simulink library "lib_all"  (Simulink 7.6)
settings: Solver=ode45  SolverMode=SingleTasking  StartTime=0.0  StopTime=10.0  RelTol=1e-3
values from neighbouring files (symbols this model uses):
  map_lib_03 = [6.8679 7.0159 7.4571 8.1849 9.1892 10.456 11.97 13.71 15.655 17.781 20.063 22.474 ... (803 elements, 73x11)]    [scr_simdata.mat]    (also in scr_simdata_all.mat)
  map_lib_06 = [2.5784 2.6727 2.9588 3.4453 4.1444 5.068 6.226 7.6242 9.2634 11.139 13.24 15.552 ... (803 elements, 73x11)]    [scr_simdata.mat]    (also in scr_simdata_all.mat)
  map_lib_09 = [0.0025784 0.0026727 0.0029588 0.0034453 0.0041444 0.005068 0.006226 0.0076242 0.0092634 0.011139 0.01324 0.015552 ... (803 elements, 73x11)]    [scr_simdata.mat]    (also in scr_simdata_all.mat)
  map_lib_10 = [2.74 2.75 2.76 2.77 2.78 2.79 2.8 2.81 2.82 2.83 2.84 2.85 ... (81 elements, 1x81)]    [scr_simdata.mat]    (also in scr_simdata_all.mat)
  map_lib_11 = [1.1983 1.2027 1.207 1.2113 1.2156 1.2198 1.2241 1.2283 1.2326 1.2368 1.241 1.2451 ... (81 elements, 1x81)]    [scr_simdata.mat]    (also in scr_simdata_all.mat)
  map_lib_12 = [2.74 2.75 2.76 2.77 2.78 2.79 2.8 2.81 2.82 2.83 2.84 2.85 ... (81 elements, 1x81)]    [scr_simdata.mat]    (also in scr_simdata_all.mat)
  map_lib_13 = [1.74 1.75 1.76 1.77 1.78 1.79 1.8 1.81 1.82 1.83 1.84 1.85 ... (81 elements, 1x81)]    [scr_simdata.mat]    (also in scr_simdata_all.mat)
  map_lib_16 = [0.00048828125 1.6159 1.8962 2.0821 2.2251 2.3426 2.4433 2.5318 2.611 2.683 2.749 2.8101 ... (1419 elements, 129x11)]    [scr_simdata.mat]    (also in scr_simdata_all.mat)
  map_mr2_01 = [0 800 1600 2400 3200 4000]    [scr_simdata.mat]    (also in scr_simdata_all.mat)
  map_mr2_02 = [1.32 1.32 1.33 1.34 1.36 1.38]    [scr_simdata.mat]    (also in scr_simdata_all.mat)
  map_mr2_03 = [0 800 1600 2400 3200 4000]    [scr_simdata.mat]    (also in scr_simdata_all.mat)
  map_mr2_04 = [1.32 1.32 1.33 1.34 1.36 1.38]    [scr_simdata.mat]    (also in scr_simdata_all.mat)
  map_mr2_05 = [0 800 1600 2400 3200 4000]    [scr_simdata.mat]    (also in scr_simdata_all.mat)
  map_mr2_06 = [1.32 1.32 1.33 1.34 1.36 1.38]    [scr_simdata.mat]    (also in scr_simdata_all.mat)
  Parameter1 = 6    [scr_simdata.mat]    (also in scr_simdata_all.mat)
  Parameter101 = 30    [scr_simdata.mat]    (also in scr_simdata_all.mat)
  Parameter102 = 12    [scr_simdata.mat]    (also in scr_simdata_all.mat)
  Parameter103 = -240    [scr_simdata.mat]    (also in scr_simdata_all.mat)
  Parameter108 = 48    [scr_simdata.mat]    (also in scr_simdata_all.mat)
  Parameter120 = 16    [scr_simdata.mat]    (also in scr_simdata_all.mat)
  Parameter121 = 87    [scr_simdata.mat]    (also in scr_simdata_all.mat)
  Parameter123 = 78    [scr_simdata.mat]    (also in scr_simdata_all.mat)
  Parameter124 = 60    [scr_simdata.mat]    (also in scr_simdata_all.mat)
  Parameter125 = -480    [scr_simdata.mat]    (also in scr_simdata_all.mat)
  Parameter130 = 96    [scr_simdata.mat]    (also in scr_simdata_all.mat)
  Parameter131 = 3    [scr_simdata.mat]    (also in scr_simdata_all.mat)
  Parameter144 = 3    [scr_simdata.mat]    (also in scr_simdata_all.mat)
  Parameter18 = 128    [scr_simdata.mat]    (also in scr_simdata_all.mat)
  Parameter19 = 16    [scr_simdata.mat]    (also in scr_simdata_all.mat)
  Parameter2 = 12    [scr_simdata.mat]    (also in scr_simdata_all.mat)
  Parameter20 = 7    [scr_simdata.mat]    (also in scr_simdata_all.mat)
  Parameter21 = 4    [scr_simdata.mat]    (also in scr_simdata_all.mat)
  Parameter3 = 18    [scr_simdata.mat]    (also in scr_simdata_all.mat)
  Parameter32 = 1    [scr_simdata.mat]    (also in scr_simdata_all.mat)
  Parameter34 = 24    [scr_simdata.mat]    (also in scr_simdata_all.mat)
  Parameter4 = 138    [scr_simdata.mat]    (also in scr_simdata_all.mat)
  Parameter42 = 48    [scr_simdata.mat]    (also in scr_simdata_all.mat)
  Parameter5 = 135    [scr_simdata.mat]    (also in scr_simdata_all.mat)
  Parameter55 = 0    [scr_simdata.mat]    (also in scr_simdata_all.mat)
  Parameter56 = 0    [scr_simdata.mat]    (also in scr_simdata_all.mat)
  Parameter57 = 1    [scr_simdata.mat]    (also in scr_simdata_all.mat)
  Parameter58 = 1    [scr_simdata.mat]    (also in scr_simdata_all.mat)
  Parameter59 = 2    [scr_simdata.mat]    (also in scr_simdata_all.mat)
  Parameter6 = 132    [scr_simdata.mat]    (also in scr_simdata_all.mat)
  Parameter60 = 2    [scr_simdata.mat]    (also in scr_simdata_all.mat)
  Parameter63 = 144    [scr_simdata.mat]    (also in scr_simdata_all.mat)
  Parameter7 = 126    [scr_simdata.mat]    (also in scr_simdata_all.mat)
  Parameter76 = 64    [scr_simdata.mat]    (also in scr_simdata_all.mat)
  Parameter77 = 135    [scr_simdata.mat]    (also in scr_simdata_all.mat)
  Parameter79 = 126    [scr_simdata.mat]    (also in scr_simdata_all.mat)
  Parameter80 = 108    [scr_simdata.mat]    (also in scr_simdata_all.mat)
  Parameter81 = 0    [scr_simdata.mat]    (also in scr_simdata_all.mat)
  Parameter86 = 0    [scr_simdata.mat]    (also in scr_simdata_all.mat)
  Parameter98 = 112    [scr_simdata.mat]    (also in scr_simdata_all.mat)
  Parameter99 = 39    [scr_simdata.mat]    (also in scr_simdata_all.mat)
undefined symbols (not defined in the file or in its folder): cylvolkp_hih_map2h, cylvolkp_map2h, pk1k_map2h
libraries: simulink x2
(part 9 of 36)

== system lib_all/SubSystem660/SubSystem686/SubSystem688 ==
  note: Text99
  note: Text98
  Block1563 : Inport
  Block1564 : Inport  Port=2
  Block1565 : Constant  Value=0  OutDataTypeStr=double  SampleTime=-1
  Block1566 : Constant  Value=0  OutDataTypeStr=double  SampleTime=-1
  Block1567 : Constant  Value=720  OutDataTypeStr=double  SampleTime=-1
  Block1568 : Constant  Value=5  OutDataTypeStr=double  SampleTime=-1
  Block1569 : DataStoreRead  DataStoreName=DSM50
  Block1570 : DataStoreRead  DataStoreName=DSM35
  Block1571 : Gain  Gain=5  SaturateOnIntegerOverflow=on  OutDataTypeStr=Inherit: Same as input  ParamDataTypeStr=Inherit: Same as input
      settings: AttributesFormatString=Attribute333
  Block1572 : Gain  Gain=5  SaturateOnIntegerOverflow=on  OutDataTypeStr=Inherit: Same as input  ParamDataTypeStr=Inherit: Same as input
      settings: AttributesFormatString=Attribute334
  Block1573 : Gain  Gain=1/5  SaturateOnIntegerOverflow=on  OutDataTypeStr=Inherit: Same as input  ParamDataTypeStr=Inherit: Same as input
      settings: AttributesFormatString=Attribute335
  Block1574 : Product  Inputs=*/  OutDataTypeStr=double  SaturateOnIntegerOverflow=on  RndMeth=Zero  InputSameDT=on
  Block1575 : Rounding
  Block1576 : Rounding
  Block1577 : Sum  Inputs=+-+  OutDataTypeStr=double  RndMeth=Zero  SaturateOnIntegerOverflow=on  InputSameDT=on
  Block1578 : Sum  Inputs=-+  OutDataTypeStr=double  RndMeth=Zero  SaturateOnIntegerOverflow=on  InputSameDT=on
  SubSystem689 : Subsystem  TreatAsAtomicUnit=on  mask(***ATC.DIVISION***){bd_version=1.01.A, r0=チェック有り, r1=, r2=, r3=, r4=, r5=, r6=, r7=, r8=, r9=, c0=, c1=, c2=, c3=, c4=, c5=, c6=, c7=, c8=, c9=}
      mask prompts: bd_version "Version"
      mask help: これはAutoCからのブロックです．編集禁止．
      settings: OpenFcn=warndlg('変更禁止!');
      mask Help: web(ui_blockhelpmng(gcb));
  SubSystem690 : Subsystem  TreatAsAtomicUnit=on  mask(***ATC.DSRVEC***){bd_version=1.01.A, r0=DSM95, r1=-1, r2=, r3=, r4=, r5=, r6=, r7=, r8=, r9=, c0=, c1=, c2=, c3=, c4=, c5=, c6=, c7=, c8=, c9=}
      mask prompts: bd_version "Version"
      mask help: これはAutoCからのブロックです．編集禁止．
      settings: OpenFcn=warndlg('変更禁止!');
      mask Help: web(ui_blockhelpmng(gcb));
      mask Display: disp('ATC.DSRVEC')
  SubSystem691 : Subsystem
      settings: RTWSystemCode=Inline
  Block1587 : Outport
  Block1588 : Outport  Port=2
  wires:
  Block1576/1 -> Block1587/1
  Block1573/1 -> Block1576/1
  Block1572/1 -> Block1577/3
  Block1569/1 -> Block1577/2
  Block1570/1 -> SubSystem689/2(div)
  SubSystem690/1(Output) -> SubSystem689/1(mult)
  Block1565/1 -> SubSystem689/3(init)
  Block1568/1 -> Block1574/2
  Block1578/1 -> Block1588/1
  Block1564/1 -> SubSystem690/1(element)
  Block1567/1 -> SubSystem691/1(Block1579)
  Block1577/1 -> SubSystem691/2(Block1580)  "SigLabel39"
  Block1566/1 -> SubSystem691/3(Block1581)
  SubSystem691/1(Block1586) -> Block1574/1, Block1573/1, Block1578/2
  Block1574/1 -> Block1575/1
  Block1575/1 -> Block1571/1
  Block1571/1 -> Block1578/1
  Block1563/1 -> Block1572/1
  SubSystem689/1(Out) -> Block1577/1  "SigLabel38"

== system lib_all/SubSystem660/SubSystem686/SubSystem694 ==
  note: Text102
  note: Text101
  note: Text100
  Block1589 : Inport
  Block1590 : Inport  Port=2
  Block1591 : Inport  Port=3
  Block1592 : Constant  Value=0  OutDataTypeStr=double  SampleTime=-1
  Block1593 : Constant  OutDataTypeStr=double  SampleTime=-1
  Block1594 : Constant  OutDataTypeStr=double  SampleTime=-1
  Block1595 : Constant  Value=0  OutDataTypeStr=double  SampleTime=-1
  Block1596 : Constant  OutDataTypeStr=double  SampleTime=-1
  Block1597 : Constant  Value=143  OutDataTypeStr=double  SampleTime=-1
  Block1598 : Demux  Outputs=2
  Block1599 : Logic  Operator=NOT
  Block1600 : Mux  Inputs=2
  Block1601 : Mux  Inputs=2
  Block1602 : Sum  OutDataTypeStr=double  RndMeth=Zero  SaturateOnIntegerOverflow=on  InputSameDT=on
  Block1603 : Sum  OutDataTypeStr=double  RndMeth=Zero  SaturateOnIntegerOverflow=on  InputSameDT=on
  Block1604 : Switch  Threshold=0.5  RndMeth=Zero  SaturateOnIntegerOverflow=on  InputSameDT=on
  Block1605 : Switch  Threshold=0.5  RndMeth=Zero  SaturateOnIntegerOverflow=on  InputSameDT=on
  Block1606 : Switch  Threshold=0.5  RndMeth=Zero  SaturateOnIntegerOverflow=on  InputSameDT=on
  SubSystem695 : Subsystem
  SubSystem696 : Subsystem
  SubSystem697 : Subsystem
  SubSystem698 : Subsystem  TreatAsAtomicUnit=on
  SubSystem707 : Subsystem  TreatAsAtomicUnit=on
  SubSystem717 : Subsystem  TreatAsAtomicUnit=on
  Block1651 : Outport
  Block1652 : Outport  Port=2
  wires:
  Block1589/1 -> SubSystem695/1(In1), Block1602/2, Block1600/1
  Block1597/1 -> SubSystem695/2(In2), Block1601/1
  Block1590/1 -> SubSystem696/1(In1), SubSystem697/1(In1)
  Block1592/1 -> SubSystem696/2(In2)
  SubSystem696/1(Out1) -> Block1604/2, SubSystem698/Enable
  SubSystem698/1(Block1620) -> Block1604/1
  Block1593/1 -> SubSystem697/2(In2)
  SubSystem697/1(Out1) -> Block1605/2, SubSystem707/Enable, Block1599/1
  SubSystem707/1(Block1635) -> Block1605/1
  Block1599/1 -> SubSystem717/Enable
  Block1605/1 -> Block1604/3
  SubSystem717/1(Block1650) -> Block1605/3
  Block1598/1 -> Block1651/1
  Block1598/2 -> Block1652/1
  Block1604/1 -> Block1598/1
  SubSystem695/1(Out1) -> Block1606/2
  Block1594/1 -> Block1602/1
  Block1595/1 -> Block1601/2
  Block1601/1 -> Block1606/3
  Block1602/1 -> Block1600/2
  Block1600/1 -> Block1606/1
  Block1591/1 -> SubSystem698/2(Block1608), SubSystem707/2(Block1622), SubSystem717/2(Block1637)
  Block1606/1 -> Block1603/2
  Block1596/1 -> Block1603/1
  Block1603/1 -> SubSystem698/1(Block1607), SubSystem707/1(Block1621), SubSystem717/1(Block1636)

== system lib_all/SubSystem7/SubSystem134/SubSystem135 ==
  note: ***編集禁止***
  In1 : Inport
  In2 : Inport  Port=2
  RO1 : RelationalOperator  Operator===  InputSameDT=on
  single1 : DataTypeConversion  OutDataTypeStr=single  SaturateOnIntegerOverflow=on  RndMeth=Zero
  single2 : DataTypeConversion  OutDataTypeStr=single  SaturateOnIntegerOverflow=on  RndMeth=Zero
  Out1 : Outport
  wires:
  RO1/1 -> Out1/1
  In2/1 -> single2/1
  In1/1 -> single1/1
  single1/1 -> RO1/1
  single2/1 -> RO1/2

== system lib_all/SubSystem7/SubSystem134/SubSystem136 ==
  note: Text21
  Block317 : Inport
  Block318 : EnablePort
  Block319 : Constant  Value=Parameter18  OutDataTypeStr=double  SampleTime=-1
  SubSystem137 : Subsystem  TreatAsAtomicUnit=on
      settings: RTWSystemCode=Inline
  SubSystem139 : Subsystem
  SubSystem149 : Subsystem  TreatAsAtomicUnit=on
  wires:
  Block317/1 -> SubSystem149/2(Block344), SubSystem139/3(Block325)
  Block319/1 -> SubSystem137/1(N)
  SubSystem137/1(action) -> SubSystem149/Trigger
  SubSystem137/2(i) -> SubSystem149/1(Block343)
  SubSystem149/1(Block361) -> SubSystem139/1(Block323)
  SubSystem149/2(Block362) -> SubSystem139/2(Block324)

== system lib_all/SubSystem7/SubSystem134/SubSystem159 ==
  note: ***編集禁止***
  element : Inport
  Inside_DSR : DataStoreRead  DataStoreName=DSM374
  Selector : Selector  IndexOptions=Index vector (port)  Indices=[1 3]  OutputSizes=1
  Output : Outport
  wires:
  Selector/1 -> Output/1
  element/1 -> Selector/2
  Inside_DSR/1 -> Selector/1

== system lib_all/SubSystem7/SubSystem134/SubSystem160 ==
  note: ***編集禁止***
  input : Inport
  element : Inport  Port=2
  Assignment : Assignment  IndexOptions=Index vector (port)  Indices=1  OutputSizes=[1 1]
  Inside_DSR : DataStoreRead  DataStoreName=DSM374
  Inside_DSW : DataStoreWrite  DataStoreName=DSM374
  wires:
  element/1 -> Assignment/3
  input/1 -> Assignment/2
  Inside_DSR/1 -> Assignment/1
  Assignment/1 -> Inside_DSW/1

== system lib_all/SubSystem7/SubSystem161/SubSystem162 ==
  note: ***編集禁止***
  In1 : Inport
  In2 : Inport  Port=2
  LO1 : Logic  Operator=OR
  RO1 : RelationalOperator  InputSameDT=on
  RO2 : RelationalOperator  Operator===  InputSameDT=on
  single1 : DataTypeConversion  OutDataTypeStr=single  SaturateOnIntegerOverflow=on  RndMeth=Zero
  single2 : DataTypeConversion  OutDataTypeStr=single  SaturateOnIntegerOverflow=on  RndMeth=Zero
  Out1 : Outport
  wires:
  In2/1 -> single2/1
  In1/1 -> single1/1
  single1/1 -> RO1/1, RO2/1
  single2/1 -> RO1/2, RO2/2
  RO1/1 -> LO1/1
  RO2/1 -> LO1/2
  LO1/1 -> Out1/1

== system lib_all/SubSystem7/SubSystem161/SubSystem163 ==
  note: ***編集禁止***
  In1 : Inport
  In2 : Inport  Port=2
  RO1 : RelationalOperator  Operator===  InputSameDT=on
  single1 : DataTypeConversion  OutDataTypeStr=single  SaturateOnIntegerOverflow=on  RndMeth=Zero
  single2 : DataTypeConversion  OutDataTypeStr=single  SaturateOnIntegerOverflow=on  RndMeth=Zero
  Out1 : Outport
  wires:
  RO1/1 -> Out1/1
  In2/1 -> single2/1
  In1/1 -> single1/1
  single1/1 -> RO1/1
  single2/1 -> RO1/2

== system lib_all/SubSystem7/SubSystem161/SubSystem164 ==
  note: Text25
  Block367 : EnablePort
  Block368 : Constant  Value=Parameter131  OutDataTypeStr=double  SampleTime=-1
  SubSystem165 : Subsystem  TreatAsAtomicUnit=on
      settings: RTWSystemCode=Inline
  SubSystem167 : Subsystem  TreatAsAtomicUnit=on
      settings: RTWSystemCode=Inline
  SubSystem169 : Subsystem  TreatAsAtomicUnit=on
  SubSystem173 : Subsystem  TreatAsAtomicUnit=on
  SubSystem175 : Subsystem  Priority=2
      settings: AttributesFormatString=Attribute80
  wires:
  Block368/1 -> SubSystem165/1(N), SubSystem167/1(N)
  SubSystem165/1(action) -> SubSystem169/Trigger
  SubSystem165/2(i) -> SubSystem169/1(Block375)
  SubSystem167/1(action) -> SubSystem173/Trigger
  SubSystem167/2(i) -> SubSystem173/1(Block379)

== system lib_all/SubSystem7/SubSystem9/SubSystem10 ==
  note: ***編集禁止***
  In1 : Inport
  In2 : Inport  Port=2
  LO1 : Logic
  RO1 : RelationalOperator  Operator=<  InputSameDT=on
  RO2 : RelationalOperator  Operator=~=  InputSameDT=on
  single1 : DataTypeConversion  OutDataTypeStr=single  SaturateOnIntegerOverflow=on  RndMeth=Zero
  single2 : DataTypeConversion  OutDataTypeStr=single  SaturateOnIntegerOverflow=on  RndMeth=Zero
  Out1 : Outport
  wires:
  In2/1 -> single2/1
  In1/1 -> single1/1
  single1/1 -> RO1/1, RO2/1
  single2/1 -> RO1/2, RO2/2
  RO1/1 -> LO1/1
  RO2/1 -> LO1/2
  LO1/1 -> Out1/1

== system lib_all/SubSystem7/SubSystem9/SubSystem11 ==
  Block16 : Inport
  Block17 : Constant  Value=Parameter120  OutDataTypeStr=double  SampleTime=-1
  Block18 : Constant  Value=Parameter80  OutDataTypeStr=double  SampleTime=-1
  Block19 : Constant  Value=Parameter102  OutDataTypeStr=double  SampleTime=-1
  Block20 : Constant  Value=Parameter124  OutDataTypeStr=double  SampleTime=-1
  Block21 : Constant  Value=Parameter76  OutDataTypeStr=double  SampleTime=-1
  Block22 : Constant  Value=Parameter98  OutDataTypeStr=double  SampleTime=-1
  Block23 : MultiPortSwitch  zeroidx=off  RndMeth=Zero  SaturateOnIntegerOverflow=on  InputSameDT=on
  Block24 : Mux  Inputs=2
  Block25 : Mux  Inputs=2
  Block26 : Mux  Inputs=2
  Block27 : Outport
  wires:
  Block23/1 -> Block27/1
  Block16/1 -> Block23/1
  Block18/1 -> Block24/1
  Block19/1 -> Block25/1
  Block20/1 -> Block26/1
  Block17/1 -> Block26/2
  Block22/1 -> Block25/2
  Block24/1 -> Block23/2
  Block26/1 -> Block23/4
  Block25/1 -> Block23/3
  Block21/1 -> Block24/2

== system lib_all/SubSystem7/SubSystem9/SubSystem12 ==
  note: ***編集禁止***
  element : Inport
  Inside_DSR : DataStoreRead  DataStoreName=DSM374
  Selector : Selector  IndexOptions=Index vector (port)  Indices=[1 3]  OutputSizes=1
  Output : Outport
  wires:
  Selector/1 -> Output/1
  element/1 -> Selector/2
  Inside_DSR/1 -> Selector/1

== system lib_all/SubSystem7/SubSystem9/SubSystem13 ==
  Block28 : Inport
  Block29 : Inport  Port=2
  Block30 : Inport  Port=3
  Block31 : Inport  Port=4
  Block32 : EnablePort
  Block33 : Constant  Value=0  OutDataTypeStr=double  SampleTime=-1
  Block34 : Constant  OutDataTypeStr=double  SampleTime=-1
  Block35 : Constant  OutDataTypeStr=double  SampleTime=-1
  Block36 : Constant  Value=0  OutDataTypeStr=double  SampleTime=-1
  Block37 : Sum  OutDataTypeStr=double  RndMeth=Zero  SaturateOnIntegerOverflow=on  InputSameDT=on
  Block38 : Sum  OutDataTypeStr=double  RndMeth=Zero  SaturateOnIntegerOverflow=on  InputSameDT=on
  SubSystem120 : Subsystem
  SubSystem127 : Subsystem
  SubSystem14 : Subsystem  TreatAsAtomicUnit=on
      settings: AttributesFormatString=Attribute16  RTWFcnNameOpts=Use subsystem name
  SubSystem67 : Subsystem  TreatAsAtomicUnit=on
      settings: AttributesFormatString=Attribute35  RTWFcnNameOpts=Use subsystem name
  wires:
  Block38/1 -> SubSystem127/3(Block299), SubSystem120/3(Block285)
  Block35/1 -> Block38/2
  Block31/1 -> Block38/1
  Block28/1 -> SubSystem67/1(Block161), SubSystem14/1(Block39), Block37/1
  Block30/1 -> SubSystem14/2(Block40)
  Block29/1 -> SubSystem67/2(Block162)
  Block36/1 -> SubSystem14/4(Block42), SubSystem14/3(Block41)
  Block33/1 -> SubSystem67/4(Block164), SubSystem67/3(Block163)
  SubSystem14/1(Block160) -> SubSystem120/1(Block283)
  Block37/1 -> SubSystem120/2(Block284), SubSystem127/2(Block298)
  SubSystem67/1(Block282) -> SubSystem127/1(Block297)
  Block34/1 -> Block37/2

== system lib_all/SubSystem727/SubSystem740/SubSystem742 ==
  note: ***編集禁止***
  In1 : Inport
  In2 : Inport  Port=2
  RO1 : RelationalOperator  Operator===  InputSameDT=on
  single1 : DataTypeConversion  OutDataTypeStr=single  SaturateOnIntegerOverflow=on  RndMeth=Zero
  single2 : DataTypeConversion  OutDataTypeStr=single  SaturateOnIntegerOverflow=on  RndMeth=Zero
  Out1 : Outport
  wires:
  RO1/1 -> Out1/1
  In2/1 -> single2/1
  In1/1 -> single1/1
  single1/1 -> RO1/1
  single2/1 -> RO1/2

== system lib_all/SubSystem727/SubSystem740/SubSystem743 ==
  note: Text110
  Block1732 : Inport
  Block1733 : Inport  Port=2
  Block1734 : Constant  Value=0  OutDataTypeStr=double  SampleTime=-1
  Block1735 : Constant  Value=720  OutDataTypeStr=double  SampleTime=-1
  Block1736 : Gain  Gain=5  SaturateOnIntegerOverflow=on  OutDataTypeStr=Inherit: Same as input  ParamDataTypeStr=Inherit: Same as input
      settings: AttributesFormatString=Attribute353
  SubSystem744 : Subsystem
      settings: RTWFcnNameOpts=Use subsystem name
  SubSystem749 : Subsystem
      settings: RTWSystemCode=Inline
  Block1762 : Outport
  wires:
  Block1736/1 -> SubSystem749/2(Block1757)
  SubSystem744/1(Block1755) -> Block1736/1
  Block1733/1 -> SubSystem744/2(Block1738)
  Block1732/1 -> SubSystem744/1(Block1737)
  SubSystem749/1(Block1761) -> Block1762/1
  Block1735/1 -> SubSystem749/1(Block1756)
  Block1734/1 -> SubSystem749/3(Block1758)

== system lib_all/SubSystem727/SubSystem740/SubSystem751 ==
  Block1763 : Inport
  Block1764 : Inport  Port=2
  Block1765 : Inport  Port=3
  Block1766 : EnablePort
  Block1767 : DataStoreWrite  DataStoreName=DSM12
  Block1768 : DataStoreWrite  DataStoreName=DSM14
  Block1769 : DataStoreWrite  DataStoreName=DSM13
  wires:
  Block1763/1 -> Block1767/1
  Block1765/1 -> Block1769/1
  Block1764/1 -> Block1768/1

== system lib_all/SubSystem727/SubSystem740/SubSystem752 ==
  note: Text111
  Block1770 : Inport
  Block1771 : Inport  Port=2
  Block1772 : Constant  Value=360  OutDataTypeStr=double  SampleTime=-1
  Block1773 : Constant  Value=360  OutDataTypeStr=double  SampleTime=-1
  Block1774 : Sum  Inputs=+-  OutDataTypeStr=double  RndMeth=Zero  SaturateOnIntegerOverflow=on  InputSameDT=on
  Block1775 : Switch  Threshold=0.5  RndMeth=Zero  SaturateOnIntegerOverflow=on  InputSameDT=on
  Block1776 : Lookup2D  RowIndex=map_lib_05  ColumnIndex=map_lib_04  Table=map_lib_06  SaturateOnIntegerOverflow=on  InputSameDT=on
      settings: AttributesFormatString=<%<OutputValues>>\nAttribute362
  SubSystem753 : Subsystem
  Block1777 : Outport
  wires:
  Block1771/1 -> SubSystem753/1(In1), Block1775/3, Block1774/1
  Block1773/1 -> SubSystem753/2(In2)
  SubSystem753/1(Out1) -> Block1775/2
  Block1774/1 -> Block1775/1
  Block1772/1 -> Block1774/2
  Block1776/1 -> Block1777/1
  Block1770/1 -> Block1776/2
  Block1775/1 -> Block1776/1  "SigLabel45"

== system lib_all/SubSystem727/SubSystem754/SubSystem756 ==
  note: Text115
  note: Text114
  Block1788 : Inport
  Block1789 : Inport  Port=2
  Block1790 : Constant  Value=0  OutDataTypeStr=double  SampleTime=-1
  Block1791 : Constant  Value=0  OutDataTypeStr=double  SampleTime=-1
  Block1792 : Constant  Value=720  OutDataTypeStr=double  SampleTime=-1
  Block1793 : Constant  Value=5  OutDataTypeStr=double  SampleTime=-1
  Block1794 : DataStoreRead  DataStoreName=DSM50
  Block1795 : DataStoreRead  DataStoreName=DSM35
  Block1796 : Gain  Gain=5  SaturateOnIntegerOverflow=on  OutDataTypeStr=Inherit: Same as input  ParamDataTypeStr=Inherit: Same as input
      settings: AttributesFormatString=Attribute370
  Block1797 : Gain  Gain=5  SaturateOnIntegerOverflow=on  OutDataTypeStr=Inherit: Same as input  ParamDataTypeStr=Inherit: Same as input
      settings: AttributesFormatString=Attribute371
  Block1798 : Gain  Gain=1/5  SaturateOnIntegerOverflow=on  OutDataTypeStr=Inherit: Same as input  ParamDataTypeStr=Inherit: Same as input
      settings: AttributesFormatString=Attribute372
  Block1799 : Product  Inputs=*/  OutDataTypeStr=double  SaturateOnIntegerOverflow=on  RndMeth=Zero  InputSameDT=on
  Block1800 : Rounding
  Block1801 : Rounding
  Block1802 : Sum  Inputs=+-+  OutDataTypeStr=double  RndMeth=Zero  SaturateOnIntegerOverflow=on  InputSameDT=on
  Block1803 : Sum  Inputs=-+  OutDataTypeStr=double  RndMeth=Zero  SaturateOnIntegerOverflow=on  InputSameDT=on
  SubSystem757 : Subsystem  TreatAsAtomicUnit=on  mask(***ATC.DIVISION***){bd_version=1.01.A, r0=チェック有り, r1=, r2=, r3=, r4=, r5=, r6=, r7=, r8=, r9=, c0=, c1=, c2=, c3=, c4=, c5=, c6=, c7=, c8=, c9=}
      mask prompts: bd_version "Version"
      mask help: これはAutoCからのブロックです．編集禁止．
      settings: OpenFcn=warndlg('変更禁止!');
      mask Help: web(ui_blockhelpmng(gcb));
  SubSystem758 : Subsystem  TreatAsAtomicUnit=on  mask(***ATC.DSRVEC***){bd_version=1.01.A, r0=DSM95, r1=-1, r2=, r3=, r4=, r5=, r6=, r7=, r8=, r9=, c0=, c1=, c2=, c3=, c4=, c5=, c6=, c7=, c8=, c9=}
      mask prompts: bd_version "Version"
      mask help: これはAutoCからのブロックです．編集禁止．
      settings: OpenFcn=warndlg('変更禁止!');
      mask Help: web(ui_blockhelpmng(gcb));
      mask Display: disp('ATC.DSRVEC')
  SubSystem759 : Subsystem
      settings: RTWSystemCode=Inline
  Block1812 : Outport
  Block1813 : Outport  Port=2
  wires:
  Block1801/1 -> Block1812/1
  Block1798/1 -> Block1801/1
  Block1797/1 -> Block1802/3
  Block1794/1 -> Block1802/2
  Block1795/1 -> SubSystem757/2(div)
  SubSystem758/1(Output) -> SubSystem757/1(mult)
  Block1790/1 -> SubSystem757/3(init)
  Block1793/1 -> Block1799/2
  Block1803/1 -> Block1813/1
  Block1789/1 -> SubSystem758/1(element)
  Block1792/1 -> SubSystem759/1(Block1804)
  Block1802/1 -> SubSystem759/2(Block1805)  "SigLabel47"
  Block1791/1 -> SubSystem759/3(Block1806)
  SubSystem759/1(Block1811) -> Block1799/1, Block1798/1, Block1803/2
  Block1799/1 -> Block1800/1
  Block1800/1 -> Block1796/1
  Block1796/1 -> Block1803/1
  Block1788/1 -> Block1797/1
  SubSystem757/1(Out) -> Block1802/1  "SigLabel46"

== system lib_all/SubSystem727/SubSystem754/SubSystem762 ==
  note: Text118
  note: Text117
  note: Text116
  Block1814 : Inport
  Block1815 : Inport  Port=2
  Block1816 : Inport  Port=3
  Block1817 : Constant  Value=0  OutDataTypeStr=double  SampleTime=-1
  Block1818 : Constant  OutDataTypeStr=double  SampleTime=-1
  Block1819 : Constant  OutDataTypeStr=double  SampleTime=-1
  Block1820 : Constant  Value=0  OutDataTypeStr=double  SampleTime=-1
  Block1821 : Constant  OutDataTypeStr=double  SampleTime=-1
  Block1822 : Constant  Value=143  OutDataTypeStr=double  SampleTime=-1
  Block1823 : Demux  Outputs=2
  Block1824 : Logic  Operator=NOT
  Block1825 : Mux  Inputs=2
  Block1826 : Mux  Inputs=2
  Block1827 : Sum  OutDataTypeStr=double  RndMeth=Zero  SaturateOnIntegerOverflow=on  InputSameDT=on
  Block1828 : Sum  OutDataTypeStr=double  RndMeth=Zero  SaturateOnIntegerOverflow=on  InputSameDT=on
  Block1829 : Switch  Threshold=0.5  RndMeth=Zero  SaturateOnIntegerOverflow=on  InputSameDT=on
  Block1830 : Switch  Threshold=0.5  RndMeth=Zero  SaturateOnIntegerOverflow=on  InputSameDT=on
  Block1831 : Switch  Threshold=0.5  RndMeth=Zero  SaturateOnIntegerOverflow=on  InputSameDT=on
  SubSystem763 : Subsystem
  SubSystem764 : Subsystem
  SubSystem765 : Subsystem
  SubSystem766 : Subsystem  TreatAsAtomicUnit=on
  SubSystem775 : Subsystem  TreatAsAtomicUnit=on
  SubSystem785 : Subsystem  TreatAsAtomicUnit=on
  Block1876 : Outport
  Block1877 : Outport  Port=2
  wires:
  Block1814/1 -> SubSystem763/1(In1), Block1827/2, Block1825/1
  Block1822/1 -> SubSystem763/2(In2), Block1826/1
  Block1815/1 -> SubSystem764/1(In1), SubSystem765/1(In1)
  Block1817/1 -> SubSystem764/2(In2)
  SubSystem764/1(Out1) -> Block1829/2, SubSystem766/Enable
  SubSystem766/1(Block1845) -> Block1829/1
  Block1818/1 -> SubSystem765/2(In2)
  SubSystem765/1(Out1) -> Block1830/2, SubSystem775/Enable, Block1824/1
  SubSystem775/1(Block1860) -> Block1830/1
  Block1824/1 -> SubSystem785/Enable
  Block1830/1 -> Block1829/3
  SubSystem785/1(Block1875) -> Block1830/3
  Block1823/1 -> Block1876/1
  Block1823/2 -> Block1877/1
  Block1829/1 -> Block1823/1
  SubSystem763/1(Out1) -> Block1831/2
  Block1819/1 -> Block1827/1
  Block1820/1 -> Block1826/2
  Block1826/1 -> Block1831/3
  Block1827/1 -> Block1825/2
  Block1825/1 -> Block1831/1
  Block1816/1 -> SubSystem766/2(Block1833), SubSystem775/2(Block1847), SubSystem785/2(Block1862)
  Block1831/1 -> Block1828/2
  Block1821/1 -> Block1828/1
  Block1828/1 -> SubSystem766/1(Block1832), SubSystem775/1(Block1846), SubSystem785/1(Block1861)

== system lib_all/SubSystem800/SubSystem803/SubSystem804 ==
  note: Text130
  Block1929 : Inport
  Block1930 : Inport  Port=2
  Block1931 : Inport  Port=3
  Block{1932,1934,1936..1937} (4 alike) : Constant  OutDataTypeStr=double  SampleTime=-1
  Block1933 : Constant  Value=0  OutDataTypeStr=double  SampleTime=-1
  Block1935 : Constant  Value=0  OutDataTypeStr=double  SampleTime=-1
  Block1943 : Sum  OutDataTypeStr=double  RndMeth=Zero  SaturateOnIntegerOverflow=on  InputSameDT=on
  Block1944 : Sum  Inputs=+++  OutDataTypeStr=double  RndMeth=Zero  SaturateOnIntegerOverflow=on  InputSameDT=on
  Block1945 : Sum  Inputs=+++  OutDataTypeStr=double  RndMeth=Zero  SaturateOnIntegerOverflow=on  InputSameDT=on
  Block1946 : Sum  Inputs=--+  OutDataTypeStr=double  RndMeth=Zero  SaturateOnIntegerOverflow=on  InputSameDT=on
  Block1947 : Sum  Inputs=--+  OutDataTypeStr=double  RndMeth=Zero  SaturateOnIntegerOverflow=on  InputSameDT=on
  Block{1948..1951} (4 alike) : Switch  Threshold=0.5  RndMeth=Zero  SaturateOnIntegerOverflow=on  InputSameDT=on
  SubSystem805 : Subsystem
  SubSystem806 : Subsystem
  SubSystem807 : Subsystem
  SubSystem808 : Subsystem
  Block1952 : Outport
  wires:
  Block1943/1 -> Block1946/3, SubSystem807/2(In2), Block1950/3, SubSystem805/2(In2), Block1944/3
  Block1949/1 -> Block1952/1
  Block1931/1 -> Block1943/2
  Block1930/1 -> Block1943/1
  Block1951/1 -> Block1949/3
  Block1937/1 -> Block1947/2
  Block1947/1 -> Block1951/1
  Block1950/1 -> SubSystem808/2(In2), Block1951/3, Block1947/3
  [TagName14] -> SubSystem808/1(In1)
  SubSystem808/1(Out1) -> Block1951/2
  [TagName14] -> Block1947/1, Block1946/1
  Block1936/1 -> Block1946/2
  Block1946/1 -> Block1950/1
  [TagName14] -> SubSystem807/1(In1)
  SubSystem807/1(Out1) -> Block1950/2
  Block1948/1 -> Block1949/1
  Block1934/1 -> Block1945/2
  Block1945/1 -> Block1948/1
  Block1935/1 -> SubSystem806/1(In1)
  SubSystem806/1(Out1) -> Block1948/2
  [TagName14] -> Block1945/1, Block1944/1
  Block1932/1 -> Block1944/2
  Block1944/1 -> SubSystem806/2(In2), Block1948/3, Block1945/3
  Block1933/1 -> SubSystem805/1(In1)
  SubSystem805/1(Out1) -> Block1949/2
  Block1929/1 -> [TagName14]

== system lib_all/SubSystem800/SubSystem803/SubSystem809 ==
  note: Text131
  Block1953 : Inport
  Block1954 : Inport  Port=2
  Block1955 : Inport  Port=3
  Block{1956,1958,1960..1961} (4 alike) : Constant  OutDataTypeStr=double  SampleTime=-1
  Block1957 : Constant  Value=0  OutDataTypeStr=double  SampleTime=-1
  Block1959 : Constant  Value=0  OutDataTypeStr=double  SampleTime=-1
  Block1967 : Sum  OutDataTypeStr=double  RndMeth=Zero  SaturateOnIntegerOverflow=on  InputSameDT=on
  Block1968 : Sum  Inputs=+++  OutDataTypeStr=double  RndMeth=Zero  SaturateOnIntegerOverflow=on  InputSameDT=on
  Block1969 : Sum  Inputs=+++  OutDataTypeStr=double  RndMeth=Zero  SaturateOnIntegerOverflow=on  InputSameDT=on
  Block1970 : Sum  Inputs=--+  OutDataTypeStr=double  RndMeth=Zero  SaturateOnIntegerOverflow=on  InputSameDT=on
  Block1971 : Sum  Inputs=--+  OutDataTypeStr=double  RndMeth=Zero  SaturateOnIntegerOverflow=on  InputSameDT=on
  Block{1972..1975} (4 alike) : Switch  Threshold=0.5  RndMeth=Zero  SaturateOnIntegerOverflow=on  InputSameDT=on
  SubSystem810 : Subsystem
  SubSystem811 : Subsystem
  SubSystem812 : Subsystem
  SubSystem813 : Subsystem
  Block1976 : Outport
  wires:
  Block1967/1 -> Block1970/3, SubSystem812/2(In2), Block1974/3, SubSystem810/2(In2), Block1968/3
  Block1973/1 -> Block1976/1
  Block1955/1 -> Block1967/2
  Block1954/1 -> Block1967/1
  Block1975/1 -> Block1973/3
  Block1961/1 -> Block1971/2
  Block1971/1 -> Block1975/1
  Block1974/1 -> SubSystem813/2(In2), Block1975/3, Block1971/3
  [TagName14] -> SubSystem813/1(In1)
  SubSystem813/1(Out1) -> Block1975/2
  [TagName14] -> Block1971/1, Block1970/1
  Block1960/1 -> Block1970/2
  Block1970/1 -> Block1974/1
  [TagName14] -> SubSystem812/1(In1)
  SubSystem812/1(Out1) -> Block1974/2
  Block1972/1 -> Block1973/1
  Block1958/1 -> Block1969/2
  Block1969/1 -> Block1972/1
  Block1959/1 -> SubSystem811/1(In1)
  SubSystem811/1(Out1) -> Block1972/2
  [TagName14] -> Block1969/1, Block1968/1
  Block1956/1 -> Block1968/2
  Block1968/1 -> SubSystem811/2(In2), Block1972/3, Block1969/3
  Block1957/1 -> SubSystem810/1(In1)
  SubSystem810/1(Out1) -> Block1973/2
  Block1953/1 -> [TagName14]

== system lib_all/SubSystem800/SubSystem803/SubSystem814 ==
  note: Text132
  Block1977 : Inport
  Block1978 : Inport  Port=2
  Block1979 : Inport  Port=3
  Block{1980,1982,1984..1985} (4 alike) : Constant  OutDataTypeStr=double  SampleTime=-1
  Block1981 : Constant  Value=0  OutDataTypeStr=double  SampleTime=-1
  Block1983 : Constant  Value=0  OutDataTypeStr=double  SampleTime=-1
  Block1991 : Sum  OutDataTypeStr=double  RndMeth=Zero  SaturateOnIntegerOverflow=on  InputSameDT=on
  Block1992 : Sum  Inputs=+++  OutDataTypeStr=double  RndMeth=Zero  SaturateOnIntegerOverflow=on  InputSameDT=on
  Block1993 : Sum  Inputs=+++  OutDataTypeStr=double  RndMeth=Zero  SaturateOnIntegerOverflow=on  InputSameDT=on
  Block1994 : Sum  Inputs=--+  OutDataTypeStr=double  RndMeth=Zero  SaturateOnIntegerOverflow=on  InputSameDT=on
  Block1995 : Sum  Inputs=--+  OutDataTypeStr=double  RndMeth=Zero  SaturateOnIntegerOverflow=on  InputSameDT=on
  Block{1996..1999} (4 alike) : Switch  Threshold=0.5  RndMeth=Zero  SaturateOnIntegerOverflow=on  InputSameDT=on
  SubSystem815 : Subsystem
  SubSystem816 : Subsystem
  SubSystem817 : Subsystem
  SubSystem818 : Subsystem
  Block2000 : Outport
  wires:
  Block1991/1 -> Block1994/3, SubSystem817/2(In2), Block1998/3, SubSystem815/2(In2), Block1992/3
  Block1997/1 -> Block2000/1
  Block1979/1 -> Block1991/2
  Block1978/1 -> Block1991/1
  Block1999/1 -> Block1997/3
  Block1985/1 -> Block1995/2
  Block1995/1 -> Block1999/1
  Block1998/1 -> SubSystem818/2(In2), Block1999/3, Block1995/3
  [TagName14] -> SubSystem818/1(In1)
  SubSystem818/1(Out1) -> Block1999/2
  [TagName14] -> Block1995/1, Block1994/1
  Block1984/1 -> Block1994/2
  Block1994/1 -> Block1998/1
  [TagName14] -> SubSystem817/1(In1)
  SubSystem817/1(Out1) -> Block1998/2
  Block1996/1 -> Block1997/1
  Block1982/1 -> Block1993/2
  Block1993/1 -> Block1996/1
  Block1983/1 -> SubSystem816/1(In1)
  SubSystem816/1(Out1) -> Block1996/2
  [TagName14] -> Block1993/1, Block1992/1
  Block1980/1 -> Block1992/2
  Block1992/1 -> SubSystem816/2(In2), Block1996/3, Block1993/3
  Block1981/1 -> SubSystem815/1(In1)
  SubSystem815/1(Out1) -> Block1997/2
  Block1977/1 -> [TagName14]

== system lib_all/SubSystem800/SubSystem819/SubSystem821 ==
  note: ***編集禁止***
  In1 : Inport
  In2 : Inport  Port=2
  RO1 : RelationalOperator  Operator===  InputSameDT=on
  single1 : DataTypeConversion  OutDataTypeStr=single  SaturateOnIntegerOverflow=on  RndMeth=Zero
  single2 : DataTypeConversion  OutDataTypeStr=single  SaturateOnIntegerOverflow=on  RndMeth=Zero
  Out1 : Outport
  wires:
  RO1/1 -> Out1/1
  In2/1 -> single2/1
  In1/1 -> single1/1
  single1/1 -> RO1/1
  single2/1 -> RO1/2

== system lib_all/SubSystem800/SubSystem819/SubSystem822 ==
  note: ***編集禁止***
  In1 : Inport
  In2 : Inport  Port=2
  RO1 : RelationalOperator  Operator===  InputSameDT=on
  single1 : DataTypeConversion  OutDataTypeStr=single  SaturateOnIntegerOverflow=on  RndMeth=Zero
  single2 : DataTypeConversion  OutDataTypeStr=single  SaturateOnIntegerOverflow=on  RndMeth=Zero
  Out1 : Outport
  wires:
  RO1/1 -> Out1/1
  In2/1 -> single2/1
  In1/1 -> single1/1
  single1/1 -> RO1/1
  single2/1 -> RO1/2

== system lib_all/SubSystem800/SubSystem819/SubSystem823 ==
  note: ***編集禁止***
  In1 : Inport
  In2 : Inport  Port=2
  RO1 : RelationalOperator  Operator===  InputSameDT=on
  single1 : DataTypeConversion  OutDataTypeStr=single  SaturateOnIntegerOverflow=on  RndMeth=Zero
  single2 : DataTypeConversion  OutDataTypeStr=single  SaturateOnIntegerOverflow=on  RndMeth=Zero
  Out1 : Outport
  wires:
  RO1/1 -> Out1/1
  In2/1 -> single2/1
  In1/1 -> single1/1
  single1/1 -> RO1/1
  single2/1 -> RO1/2

== system lib_all/SubSystem800/SubSystem819/SubSystem824 ==
  note: ***編集禁止***
  In1 : Inport
  In2 : Inport  Port=2
  RO1 : RelationalOperator  Operator===  InputSameDT=on
  single1 : DataTypeConversion  OutDataTypeStr=single  SaturateOnIntegerOverflow=on  RndMeth=Zero
  single2 : DataTypeConversion  OutDataTypeStr=single  SaturateOnIntegerOverflow=on  RndMeth=Zero
  Out1 : Outport
  wires:
  RO1/1 -> Out1/1
  In2/1 -> single2/1
  In1/1 -> single1/1
  single1/1 -> RO1/1
  single2/1 -> RO1/2

== system lib_all/SubSystem800/SubSystem819/SubSystem825 ==
  note: ***編集禁止***
  In1 : Inport
  In2 : Inport  Port=2
  RO1 : RelationalOperator  Operator===  InputSameDT=on
  single1 : DataTypeConversion  OutDataTypeStr=single  SaturateOnIntegerOverflow=on  RndMeth=Zero
  single2 : DataTypeConversion  OutDataTypeStr=single  SaturateOnIntegerOverflow=on  RndMeth=Zero
  Out1 : Outport
  wires:
  RO1/1 -> Out1/1
  In2/1 -> single2/1
  In1/1 -> single1/1
  single1/1 -> RO1/1
  single2/1 -> RO1/2

== system lib_all/SubSystem800/SubSystem819/SubSystem826 ==
  note: ***編集禁止***
  In1 : Inport
  In2 : Inport  Port=2
  RO1 : RelationalOperator  Operator===  InputSameDT=on
  single1 : DataTypeConversion  OutDataTypeStr=single  SaturateOnIntegerOverflow=on  RndMeth=Zero
  single2 : DataTypeConversion  OutDataTypeStr=single  SaturateOnIntegerOverflow=on  RndMeth=Zero
  Out1 : Outport
  wires:
  RO1/1 -> Out1/1
  In2/1 -> single2/1
  In1/1 -> single1/1
  single1/1 -> RO1/1
  single2/1 -> RO1/2

== system lib_all/SubSystem800/SubSystem819/SubSystem827 ==
  Block2033 : Inport
  Block2034 : Inport  Port=2
  Block2035 : Inport  Port=3
  Block2036 : EnablePort
  Block2037 : DataStoreWrite  DataStoreName=DSM20
  Block2038 : DataStoreWrite  DataStoreName=DSM30
  Block2039 : DataStoreWrite  DataStoreName=DSM25
  wires:
  Block2035/1 -> Block2039/1
  Block2034/1 -> Block2038/1
  Block2033/1 -> Block2037/1

== system lib_all/SubSystem800/SubSystem819/SubSystem828 ==
  Block2040 : Inport
  Block2041 : Inport  Port=2
  Block2042 : Inport  Port=3
  Block2043 : EnablePort
  Block2044 : DataStoreWrite  DataStoreName=DSM21
  Block2045 : DataStoreWrite  DataStoreName=DSM31
  Block2046 : DataStoreWrite  DataStoreName=DSM26
  wires:
  Block2042/1 -> Block2046/1
  Block2041/1 -> Block2045/1
  Block2040/1 -> Block2044/1

== system lib_all/SubSystem800/SubSystem819/SubSystem829 ==
  Block2047 : Inport
  Block2048 : Inport  Port=2
  Block2049 : Inport  Port=3
  Block2050 : EnablePort
  Block2051 : DataStoreWrite  DataStoreName=DSM22
  Block2052 : DataStoreWrite  DataStoreName=DSM32
  Block2053 : DataStoreWrite  DataStoreName=DSM27
  wires:
  Block2049/1 -> Block2053/1
  Block2048/1 -> Block2052/1
  Block2047/1 -> Block2051/1

== system lib_all/SubSystem800/SubSystem819/SubSystem830 ==
  Block2054 : Inport
  Block2055 : Inport  Port=2
  Block2056 : Inport  Port=3
  Block2057 : EnablePort
  Block2058 : DataStoreWrite  DataStoreName=DSM23
  Block2059 : DataStoreWrite  DataStoreName=DSM33
  Block2060 : DataStoreWrite  DataStoreName=DSM28
  wires:
  Block2056/1 -> Block2060/1
  Block2055/1 -> Block2059/1
  Block2054/1 -> Block2058/1

== system lib_all/SubSystem800/SubSystem819/SubSystem831 ==
  Block2061 : Inport
  Block2062 : Inport  Port=2
  Block2063 : Inport  Port=3
  Block2064 : EnablePort
  Block2065 : DataStoreWrite  DataStoreName=DSM24
  Block2066 : DataStoreWrite  DataStoreName=DSM34
  Block2067 : DataStoreWrite  DataStoreName=DSM29
  wires:
  Block2061/1 -> Block2065/1
  Block2062/1 -> Block2066/1
  Block2063/1 -> Block2067/1

== system lib_all/SubSystem800/SubSystem819/SubSystem832 ==
  note: Text141
  note: Text140
  Block2068 : Inport
  Block2069 : Inport  Port=2
  Block2070 : Inport  Port=3
  Block2071 : Constant  Value=0  OutDataTypeStr=double  SampleTime=-1
  SubSystem834 : Subsystem
  SubSystem835 : Subsystem
  SubSystem843 : Subsystem  TreatAsAtomicUnit=on
  SubSystem844 : Subsystem
  Block2126 : Outport
  wires:
  [TagName23] -> SubSystem843/3(Block2113)
  [TagName11] -> SubSystem843/2(Block2112)
  [TagName1] -> SubSystem843/1(Block2111)
  SubSystem834/1(Out1) -> SubSystem843/Enable
  Block2071/1 -> SubSystem834/2(In2)
  [TagName4] -> SubSystem834/1(In1)
  SubSystem844/1(Block2125) -> Block2126/1, [TagName23]
  Block2070/1 -> SubSystem844/1(Block2118)
  SubSystem835/1(Block2110) -> SubSystem844/2(Block2119), [TagName11]
  Block2068/1 -> SubSystem835/2(Block2081), [TagName4]
  Block2069/1 -> SubSystem835/1(Block2080), [TagName1]

== system lib_all/SubSystem800/SubSystem819/SubSystem846 ==
  note: Text149
  note: Text148
  note: Text147
  Block2127 : Inport
  Block2128 : Inport  Port=2
  Block2129 : Inport  Port=3
  Block2130 : Constant  OutDataTypeStr=double  SampleTime=-1
  Block2131 : Gain  Gain=1/5  SaturateOnIntegerOverflow=on  OutDataTypeStr=Inherit: Same as input  ParamDataTypeStr=Inherit: Same as input
      settings: AttributesFormatString=Attribute440
  Block2132 : Product  OutDataTypeStr=double  SaturateOnIntegerOverflow=on  RndMeth=Zero  InputSameDT=on
  Block2133 : Sum  Inputs=-+  OutDataTypeStr=double  RndMeth=Zero  SaturateOnIntegerOverflow=on  InputSameDT=on
  Block2134 : Sum  OutDataTypeStr=double  RndMeth=Zero  SaturateOnIntegerOverflow=on  InputSameDT=on
  Block2135 : Sum  OutDataTypeStr=double  RndMeth=Zero  SaturateOnIntegerOverflow=on  InputSameDT=on
  SubSystem848 : Subsystem
  SubSystem854 : Subsystem
  Block2226 : Outport
  wires:
  Block2135/1 -> SubSystem854/3(Block2164), SubSystem848/2(Block2137)
  Block2127/1 -> Block2135/1
  Block2131/1 -> Block2134/2
  Block2132/1 -> Block2131/1
  Block2130/1 -> Block2135/2
  Block2134/1 -> Block2226/1
  SubSystem848/2(Block2161) -> Block2132/2
  Block2133/1 -> Block2132/1  "SigLabel59"
  SubSystem854/2(Block2225) -> Block2133/2
  SubSystem854/1(Block2224) -> Block2133/1, Block2134/1
  Block2129/1 -> SubSystem854/2(Block2163)
  SubSystem848/1(Block2160) -> SubSystem854/1(Block2162)
  Block2128/1 -> SubSystem848/1(Block2136)

== system lib_all/SubSystem800/SubSystem887/SubSystem889 ==
  note: ***編集禁止***
  In1 : Inport
  In2 : Inport  Port=2
  RO1 : RelationalOperator  Operator===  InputSameDT=on
  single1 : DataTypeConversion  OutDataTypeStr=single  SaturateOnIntegerOverflow=on  RndMeth=Zero
  single2 : DataTypeConversion  OutDataTypeStr=single  SaturateOnIntegerOverflow=on  RndMeth=Zero
  Out1 : Outport
  wires:
  RO1/1 -> Out1/1
  In2/1 -> single2/1
  In1/1 -> single1/1
  single1/1 -> RO1/1
  single2/1 -> RO1/2

== system lib_all/SubSystem800/SubSystem887/SubSystem890 ==
  note: ***編集禁止***
  In1 : Inport
  In2 : Inport  Port=2
  RO1 : RelationalOperator  Operator===  InputSameDT=on
  single1 : DataTypeConversion  OutDataTypeStr=single  SaturateOnIntegerOverflow=on  RndMeth=Zero
  single2 : DataTypeConversion  OutDataTypeStr=single  SaturateOnIntegerOverflow=on  RndMeth=Zero
  Out1 : Outport
  wires:
  RO1/1 -> Out1/1
  In2/1 -> single2/1
  In1/1 -> single1/1
  single1/1 -> RO1/1
  single2/1 -> RO1/2

== system lib_all/SubSystem800/SubSystem887/SubSystem891 ==
  note: ***編集禁止***
  In1 : Inport
  In2 : Inport  Port=2
  RO1 : RelationalOperator  Operator===  InputSameDT=on
  single1 : DataTypeConversion  OutDataTypeStr=single  SaturateOnIntegerOverflow=on  RndMeth=Zero
  single2 : DataTypeConversion  OutDataTypeStr=single  SaturateOnIntegerOverflow=on  RndMeth=Zero
  Out1 : Outport
  wires:
  RO1/1 -> Out1/1
  In2/1 -> single2/1
  In1/1 -> single1/1
  single1/1 -> RO1/1
  single2/1 -> RO1/2

== system lib_all/SubSystem800/SubSystem887/SubSystem892 ==
  note: ***編集禁止***
  In1 : Inport
  In2 : Inport  Port=2
  RO1 : RelationalOperator  Operator===  InputSameDT=on
  single1 : DataTypeConversion  OutDataTypeStr=single  SaturateOnIntegerOverflow=on  RndMeth=Zero
  single2 : DataTypeConversion  OutDataTypeStr=single  SaturateOnIntegerOverflow=on  RndMeth=Zero
  Out1 : Outport
  wires:
  RO1/1 -> Out1/1
  In2/1 -> single2/1
  In1/1 -> single1/1
  single1/1 -> RO1/1
  single2/1 -> RO1/2

== system lib_all/SubSystem800/SubSystem887/SubSystem893 ==
  note: ***編集禁止***
  In1 : Inport
  In2 : Inport  Port=2
  RO1 : RelationalOperator  Operator===  InputSameDT=on
  single1 : DataTypeConversion  OutDataTypeStr=single  SaturateOnIntegerOverflow=on  RndMeth=Zero
  single2 : DataTypeConversion  OutDataTypeStr=single  SaturateOnIntegerOverflow=on  RndMeth=Zero
  Out1 : Outport
  wires:
  RO1/1 -> Out1/1
  In2/1 -> single2/1
  In1/1 -> single1/1
  single1/1 -> RO1/1
  single2/1 -> RO1/2

== system lib_all/SubSystem800/SubSystem887/SubSystem894 ==
  note: ***編集禁止***
  In1 : Inport
  In2 : Inport  Port=2
  RO1 : RelationalOperator  Operator===  InputSameDT=on
  single1 : DataTypeConversion  OutDataTypeStr=single  SaturateOnIntegerOverflow=on  RndMeth=Zero
  single2 : DataTypeConversion  OutDataTypeStr=single  SaturateOnIntegerOverflow=on  RndMeth=Zero
  Out1 : Outport
  wires:
  RO1/1 -> Out1/1
  In2/1 -> single2/1
  In1/1 -> single1/1
  single1/1 -> RO1/1
  single2/1 -> RO1/2

== system lib_all/SubSystem800/SubSystem887/SubSystem895 ==
  Block2258 : Inport
  Block2259 : Inport  Port=2
  Block2260 : Inport  Port=3
  Block2261 : EnablePort
  Block2262 : DataStoreWrite  DataStoreName=DSM20
  Block2263 : DataStoreWrite  DataStoreName=DSM30
  Block2264 : DataStoreWrite  DataStoreName=DSM25
  wires:
  Block2260/1 -> Block2264/1
  Block2259/1 -> Block2263/1
  Block2258/1 -> Block2262/1

== system lib_all/SubSystem800/SubSystem887/SubSystem896 ==
  Block2265 : Inport
  Block2266 : Inport  Port=2
  Block2267 : Inport  Port=3
  Block2268 : EnablePort
  Block2269 : DataStoreWrite  DataStoreName=DSM21
  Block2270 : DataStoreWrite  DataStoreName=DSM31
  Block2271 : DataStoreWrite  DataStoreName=DSM26
  wires:
  Block2267/1 -> Block2271/1
  Block2266/1 -> Block2270/1
  Block2265/1 -> Block2269/1

== system lib_all/SubSystem800/SubSystem887/SubSystem897 ==
  Block2272 : Inport
  Block2273 : Inport  Port=2
  Block2274 : Inport  Port=3
  Block2275 : EnablePort
  Block2276 : DataStoreWrite  DataStoreName=DSM22
  Block2277 : DataStoreWrite  DataStoreName=DSM32
  Block2278 : DataStoreWrite  DataStoreName=DSM27
  wires:
  Block2274/1 -> Block2278/1
  Block2273/1 -> Block2277/1
  Block2272/1 -> Block2276/1

== system lib_all/SubSystem800/SubSystem887/SubSystem898 ==
  Block2279 : Inport
  Block2280 : Inport  Port=2
  Block2281 : Inport  Port=3
  Block2282 : EnablePort
  Block2283 : DataStoreWrite  DataStoreName=DSM23
  Block2284 : DataStoreWrite  DataStoreName=DSM33
  Block2285 : DataStoreWrite  DataStoreName=DSM28
  wires:
  Block2281/1 -> Block2285/1
  Block2280/1 -> Block2284/1
  Block2279/1 -> Block2283/1

== system lib_all/SubSystem800/SubSystem887/SubSystem899 ==
  Block2286 : Inport
  Block2287 : Inport  Port=2
  Block2288 : Inport  Port=3
  Block2289 : EnablePort
  Block2290 : DataStoreWrite  DataStoreName=DSM24
  Block2291 : DataStoreWrite  DataStoreName=DSM34
  Block2292 : DataStoreWrite  DataStoreName=DSM29
  wires:
  Block2286/1 -> Block2290/1
  Block2287/1 -> Block2291/1
  Block2288/1 -> Block2292/1

== system lib_all/SubSystem800/SubSystem887/SubSystem900 ==
  note: Text160
  note: Text159
  Block2293 : Inport
  Block2294 : Inport  Port=2
  Block2295 : Inport  Port=3
  Block2296 : Constant  Value=0  OutDataTypeStr=double  SampleTime=-1
  SubSystem902 : Subsystem
  SubSystem903 : Subsystem
  SubSystem911 : Subsystem  TreatAsAtomicUnit=on
  SubSystem912 : Subsystem
  Block2351 : Outport
  wires:
  [TagName23] -> SubSystem911/3(Block2338)
  [TagName11] -> SubSystem911/2(Block2337)
  [TagName1] -> SubSystem911/1(Block2336)
  SubSystem902/1(Out1) -> SubSystem911/Enable
  Block2296/1 -> SubSystem902/2(In2)
  [TagName4] -> SubSystem902/1(In1)
  SubSystem912/1(Block2350) -> Block2351/1, [TagName23]
  Block2295/1 -> SubSystem912/1(Block2343)
  SubSystem903/1(Block2335) -> SubSystem912/2(Block2344), [TagName11]
  Block2293/1 -> SubSystem903/2(Block2306), [TagName4]
  Block2294/1 -> SubSystem903/1(Block2305), [TagName1]

== system lib_all/SubSystem800/SubSystem887/SubSystem914 ==
  note: Text168
  note: Text167
  note: Text166
  Block2352 : Inport
  Block2353 : Inport  Port=2
  Block2354 : Inport  Port=3
  Block2355 : Constant  OutDataTypeStr=double  SampleTime=-1
  Block2356 : Gain  Gain=1/5  SaturateOnIntegerOverflow=on  OutDataTypeStr=Inherit: Same as input  ParamDataTypeStr=Inherit: Same as input
      settings: AttributesFormatString=Attribute477
  Block2357 : Product  OutDataTypeStr=double  SaturateOnIntegerOverflow=on  RndMeth=Zero  InputSameDT=on
  Block2358 : Sum  Inputs=-+  OutDataTypeStr=double  RndMeth=Zero  SaturateOnIntegerOverflow=on  InputSameDT=on
  Block2359 : Sum  OutDataTypeStr=double  RndMeth=Zero  SaturateOnIntegerOverflow=on  InputSameDT=on
  Block2360 : Sum  OutDataTypeStr=double  RndMeth=Zero  SaturateOnIntegerOverflow=on  InputSameDT=on
  SubSystem916 : Subsystem
  SubSystem922 : Subsystem
  Block2451 : Outport
  wires:
  Block2360/1 -> SubSystem922/3(Block2389), SubSystem916/2(Block2362)
  Block2352/1 -> Block2360/1
  Block2356/1 -> Block2359/2
  Block2357/1 -> Block2356/1
  Block2355/1 -> Block2360/2
  Block2359/1 -> Block2451/1
  SubSystem916/2(Block2386) -> Block2357/2
  Block2358/1 -> Block2357/1  "SigLabel69"
  SubSystem922/2(Block2450) -> Block2358/2
  SubSystem922/1(Block2449) -> Block2358/1, Block2359/1
  Block2354/1 -> SubSystem922/2(Block2388)
  SubSystem916/1(Block2385) -> SubSystem922/1(Block2387)
  Block2353/1 -> SubSystem916/1(Block2361)

== system lib_all/SubSystem800/SubSystem955/SubSystem956 ==
  note: ***編集禁止***
  input : Inport
  element : Inport  Port=2
  Assignment : Assignment  IndexOptions=Index vector (port)  Indices=1  OutputSizes=[1 1]
  Inside_DSR : DataStoreRead  DataStoreName=DSM182
  Inside_DSW : DataStoreWrite  DataStoreName=DSM182
  wires:
  element/1 -> Assignment/3
  input/1 -> Assignment/2
  Inside_DSR/1 -> Assignment/1
  Assignment/1 -> Inside_DSW/1

== system lib_all/SubSystem800/SubSystem955/SubSystem957 ==
  note: ***編集禁止***
  input : Inport
  element : Inport  Port=2
  Assignment : Assignment  IndexOptions=Index vector (port)  Indices=1  OutputSizes=[1 1]
  Inside_DSR : DataStoreRead  DataStoreName=DSM184
  Inside_DSW : DataStoreWrite  DataStoreName=DSM184
  wires:
  Assignment/1 -> Inside_DSW/1
  Inside_DSR/1 -> Assignment/1
  input/1 -> Assignment/2
  element/1 -> Assignment/3
